simulink model "TosAeb"  (R2021a)
settings: Solver=FixedStepDiscrete  StartTime=0.0  StopTime=inf  FixedStep=0.01  RelTol=1e-3
undefined symbols (not defined in the file or in its folder): ACCModSts, AccSts1, AEB_ObjectMovingOrientation, AEB_ObjectMovingState, CN_BTCActive, CN_CurrentGearR, CN_CurrentGearR_DHT, CN_PTCActive, CN_VDCActive, CN_VSC_EspOff, CN_VSC_FCW_AEB_Error, CN_VSC_FCW_AEB_NoDispay, CN_VSC_FCW_AEB_PerfmDgdtn, CN_VSC_FCW_AEB_TempUnAvlbl, CN_VSC_VehStandstill, CNaccelAvoidMax, CNaccelAvoidMin, CNbrakePedalPress, CNcomfortStrLeftAccelMax, CNcomfortStrLeftAccelMin, CNcomfortStrRightAccelMax, CNcomfortStrRightAccelMin, CNDrvDoorOpen, CNDrvModeEco, CNDrvModeNorm, CNDrvModeOther, CNDrvModeSport, CNDrvSBRreminder, CNNull, CNobjInvalidID, CNPassDoorOpen, CNPassSBRreminder, CNPEBSAccelNegInf, CNPEBSAccelNull, CNPEBSTimeInf, CNPEBSTimeNull, CNSTOPMAX, CNSTOPNULL, CNsubjWidth, CNSwtOFF, CNSwtON, CNTTCMAX, CNTTCNULL, CNVehConfigAEBCycNone, CNVehConfigAEBNone, CNVehConfigAEBPedNone, CNVehConfigFCWNone, CNVehConfigJANone, CollisionSeverity, FALSE, FCWSnvty, JACycObjCalcInfoDefaultParams, JAPedObjCalcInfoDefaultParams, JAVehObjCalcInfoDefaultParams, latCycObjCalcInfoDefaultParams, latPedObjCalcInfoDefaultParams, longObjCalcInfoDefaultParams, ModSts, movingStatus, ObjectType ... (+1112)
libraries: simulink x3031, AEBSFunctionLib x50, Ta_Library x33, AEBSStateMachineLib x23, Mylibrary x12, simulink_extras x7, ObjSelect_lib x3, dspstat3 x2
(part 16 of 102)

== system TosAeb/FcwAebJa_Step_sys/ALG_AEBSystem/ALG_AEBController/AEB_LatObjInfoCalcApp/LatCycObjCalc/LatCycObjCalc ==
  latCycObjInfo : Inport  OutDataTypeStr=Bus: BusAEBLatObj  PortDimensions=1  Partition=on  PartitionDimension=1  PartitionWidth=1  PartitionOffset=0  BusOutputAsStruct=on
  subjInfo : Inport  Port=2  OutDataTypeStr=Bus: BusSubjInfo  PortDimensions=1
  Bus Selector : BusSelector  OutputSignals=subjVel
  Compare To Constant : simulink/Logic and Bit Operations/Compare To Constant  relop===  const=FALSE  OutDataTypeStr=boolean
  For Each : ForEach  DisableCoverage=on
  If : If  IfExpression=u1
  Merge : Merge
  TgtPreSelectCalc : Subsystem  TreatAsAtomicUnit=on
  latCycObjInfoCalc : Subsystem  TreatAsAtomicUnit=on
  latCycObjInfoDefault : Subsystem  TreatAsAtomicUnit=on
  latCycCalcInfo : Outport  OutDataTypeStr=Bus: BusLatCycObjCalcInfo  ConcatenationDimension=1
  ObjPreSelectCond : Outport  Port=2  ConcatenationDimension=1
  wires:
  latCycObjInfoDefault/1(latCycCalcInfo) -> Merge/1
  latCycObjInfo/1 -> TgtPreSelectCalc/1(latCycObjInfo), latCycObjInfoCalc/2(latCycObjInfo)  "latCycObjInfo"
  subjInfo/1 -> Bus Selector/1, latCycObjInfoCalc/1(subjInfo)
  If/2 -> latCycObjInfoCalc/Action
  If/1 -> latCycObjInfoDefault/Action
  latCycObjInfoCalc/1(latCycCalcInfo) -> Merge/2
  Merge/1 -> latCycCalcInfo/1
  Bus Selector/1 -> TgtPreSelectCalc/2(subjVel)  "<subjVel>"
  TgtPreSelectCalc/1(ObjPreSelectCond) -> ObjPreSelectCond/1, Compare To Constant/1
  Compare To Constant/1 -> If/1

== system TosAeb/FcwAebJa_Step_sys/ALG_AEBSystem/ALG_AEBController/AEB_LatObjInfoCalcApp/LatCycObjCalc/TargetSelectCalc ==
  latCycCalcInfo : Inport
  ObjPreSelectCond : Inport  Port=2
  Bus Selector : BusSelector  OutputSignals=latCycObjRawInfo.ObjectID
  P_LatCycCA_SelectObjCollisionRiskProbThres : Constant  Value=P_AEB_LatCycCA_SelectObjCollisionRiskProbThres
  P_LatCycCA_SelectTgtCollisionRiskProbThres : Constant  Value=P_AEB_LatCycCA_SelectTgtCollisionRiskProbThres
  Terminator : Terminator
  Terminator1 : Terminator
  Unit Delay : UnitDelay  SampleTime=-1  HasFrameUpgradeWarning=on
      settings: AttributesFormatString=InitialCondition:%<InitialCondition>
  tgtObjFlow1 : Stateflow chart  TreatAsAtomicUnit=on
  latCycObjCalcInfo : Outport
  wires:
  Unit Delay/1 -> tgtObjFlow1/5(TargetIDK1)
  tgtObjFlow1/2(tgtIsFind) -> Terminator/1
  tgtObjFlow1/1(tgtObj) -> Bus Selector/1, latCycObjCalcInfo/1  "latCycObjCalcInfo"
  Bus Selector/1 -> Unit Delay/1  "<ObjectID>"
  ObjPreSelectCond/1 -> tgtObjFlow1/2(ObjPreSelectCondFufilled)
  P_LatCycCA_SelectObjCollisionRiskProbThres/1 -> tgtObjFlow1/3(P_LatCycCA_SelectObjCollisionRiskProbThres)
  P_LatCycCA_SelectTgtCollisionRiskProbThres/1 -> tgtObjFlow1/4(P_LatCycCA_SelectTgtCollisionRiskProbThres)
  latCycCalcInfo/1 -> tgtObjFlow1/1(latCycCalcInfo)
  tgtObjFlow1/3(tgtIndex) -> Terminator1/1

== system TosAeb/FcwAebJa_Step_sys/ALG_AEBSystem/ALG_AEBController/AEB_LatObjInfoCalcApp/LatPedObjCalc/LatPedCollisonAssessInfo ==
  ObjectID : Inport
  pedColliisonRisk : Inport  Port=2  OutDataTypeStr=single
  dxFrontBumper : Inport  Port=3  OutDataTypeStr=single
  vx : Inport  Port=4
  subjVel : Inport  Port=5
  subLongAccel : Inport  Port=6  OutDataTypeStr=single
  FcwHMISetting : Inport  Port=7  OutDataTypeStr=Enum: FCWSnvty
  If : If  IfExpression=u1
  LatPedCollisonAssessInfo : Subsystem  TreatAsAtomicUnit=on
  LatPedCollisonAssessInfoDefault : Subsystem  TreatAsAtomicUnit=on
  Merge : Merge
  ObjIsInvalidJudge : Subsystem
  latPedCollisonAssessInfo : Outport
  wires:
  ObjIsInvalidJudge/1(ObjIsInvalid) -> If/1
  If/1 -> LatPedCollisonAssessInfoDefault/Action
  If/2 -> LatPedCollisonAssessInfo/Action
  ObjectID/1 -> LatPedCollisonAssessInfo/1(ObjectID), ObjIsInvalidJudge/1(ObjectID)
  pedColliisonRisk/1 -> LatPedCollisonAssessInfo/2(pedColliisonRisk)
  dxFrontBumper/1 -> LatPedCollisonAssessInfo/3(dxFrontBumper)
  subLongAccel/1 -> LatPedCollisonAssessInfo/6(subLongAccel)
  subjVel/1 -> LatPedCollisonAssessInfo/5(subjVel)
  FcwHMISetting/1 -> LatPedCollisonAssessInfo/7(FcwHMISetting)
  LatPedCollisonAssessInfo/1(latPedCollisonAssessInfo) -> Merge/2
  Merge/1 -> latPedCollisonAssessInfo/1
  LatPedCollisonAssessInfoDefault/1(latPedCollisonAssessInfo) -> Merge/1
  vx/1 -> LatPedCollisonAssessInfo/4(vx)

== system TosAeb/FcwAebJa_Step_sys/ALG_AEBSystem/ALG_AEBController/AEB_LatObjInfoCalcApp/LatPedObjCalc/LatPedObjCalc ==
  latPedObjInfo : Inport  OutDataTypeStr=Bus: BusAEBLatObj  PortDimensions=1  Partition=on  PartitionDimension=1  PartitionWidth=1  PartitionOffset=0  BusOutputAsStruct=on
  subjInfo : Inport  Port=2  OutDataTypeStr=Bus: BusSubjInfo  PortDimensions=1
  Bus Selector : BusSelector  OutputSignals=subjVel
  Compare To Constant : simulink/Logic and Bit Operations/Compare To Constant  relop===  const=FALSE  OutDataTypeStr=boolean
  For Each : ForEach  DisableCoverage=on
  If : If  IfExpression=u1
  Merge : Merge
  ObjPreSelectCondCalc : Subsystem
  latPedObjInfoCalc : Subsystem  TreatAsAtomicUnit=on
  latPedObjInfoDefault : Subsystem  TreatAsAtomicUnit=on
  latPedCalcInfo : Outport  OutDataTypeStr=Bus: BusLatPedObjCalcInfo  ConcatenationDimension=1
  ObjPreSelectCond : Outport  Port=2  ConcatenationDimension=1
  wires:
  latPedObjInfoCalc/1(latPedCalcInfo) -> Merge/2
  If/1 -> latPedObjInfoDefault/Action
  latPedObjInfo/1 -> latPedObjInfoCalc/2(latPedObjInfo), ObjPreSelectCondCalc/1(latPedObjInfo)  "latPedObjInfo"
  If/2 -> latPedObjInfoCalc/Action
  latPedObjInfoDefault/1(latPedCalcInfo) -> Merge/1
  Merge/1 -> latPedCalcInfo/1
  subjInfo/1 -> Bus Selector/1, latPedObjInfoCalc/1(subjInfo)  "subjInfo"
  Bus Selector/1 -> ObjPreSelectCondCalc/2(subjVel)  "<subjVel>"
  ObjPreSelectCondCalc/1(ObjPreSelectCond) -> ObjPreSelectCond/1, Compare To Constant/1
  Compare To Constant/1 -> If/1

== system TosAeb/FcwAebJa_Step_sys/ALG_AEBSystem/ALG_AEBController/AEB_LatObjInfoCalcApp/LatPedObjCalc/Subsystem ==
  latPedObjInfo : Inport  OutDataTypeStr=Bus: BusAEBLatObj  PortDimensions=1  BusOutputAsStruct=on
  subjInfo : Inport  Port=2  OutDataTypeStr=Bus: BusSubjInfo  PortDimensions=1
  Bus Selector : BusSelector  OutputSignals=subjVel
  Compare To Constant : simulink/Logic and Bit Operations/Compare To Constant  relop===  const=FALSE  OutDataTypeStr=boolean
  If : If  IfExpression=u1
  Merge : Merge
  ObjPreSelectCondCalc : Subsystem
  latPedObjInfoCalc : Subsystem  TreatAsAtomicUnit=on
  latPedObjInfoDefault : Subsystem  TreatAsAtomicUnit=on
  latPedCalcInfo : Outport  OutDataTypeStr=Bus: BusLatPedObjCalcInfo
  ObjPreSelectCond : Outport  Port=2
  wires:
  latPedObjInfoCalc/1(latPedCalcInfo) -> Merge/2
  Merge/1 -> latPedCalcInfo/1
  If/2 -> latPedObjInfoCalc/Action
  subjInfo/1 -> Bus Selector/1, latPedObjInfoCalc/1(subjInfo)  "subjInfo"
  latPedObjInfo/1 -> latPedObjInfoCalc/2(latPedObjInfo), ObjPreSelectCondCalc/1(latPedObjInfo)  "latPedObjInfo"
  ObjPreSelectCondCalc/1(ObjPreSelectCond) -> ObjPreSelectCond/1, Compare To Constant/1
  Bus Selector/1 -> ObjPreSelectCondCalc/2(subjVel)  "<subjVel>"
  latPedObjInfoDefault/1(latPedCalcInfo) -> Merge/1
  Compare To Constant/1 -> If/1
  If/1 -> latPedObjInfoDefault/Action

== system TosAeb/FcwAebJa_Step_sys/ALG_AEBSystem/ALG_AEBController/AEB_LatObjInfoCalcApp/LatPedObjCalc/TargetSelectCalc ==
  latPedCalcInfo : Inport  OutDataTypeStr=Bus: BusLatPedObjCalcInfo
  ObjPreSelectCond : Inport  Port=2
  Bus Selector1 : BusSelector  OutputSignals=latPedObjRawInfo.ObjectID
  P_LatPedCA_SelectObjCollisionRiskProbThres : Constant  Value=P_AEB_LatPedCA_SelectObjCollisionRiskProbThres
  P_LatPedCA_SelectTgtCollisionRiskProbThres : Constant  Value=P_AEB_LatPedCA_SelectTgtCollisionRiskProbThres
  Terminator1 : Terminator
  Terminator2 : Terminator
  Unit Delay2 : UnitDelay  SampleTime=-1  HasFrameUpgradeWarning=on
      settings: AttributesFormatString=InitialCondition:%<InitialCondition>
  tgtObjFlow1 : Stateflow chart  TreatAsAtomicUnit=on
  latPedObjCalcInfo : Outport
  wires:
  tgtObjFlow1/1(tgtObj) -> Bus Selector1/1, latPedObjCalcInfo/1
  ObjPreSelectCond/1 -> tgtObjFlow1/2(ObjPreSelectCondFufilled)
  tgtObjFlow1/3(tgtIsFind) -> Terminator1/1
  Bus Selector1/1 -> Unit Delay2/1  "<ObjectID>"
  Unit Delay2/1 -> tgtObjFlow1/5(TargetIDK1)
  latPedCalcInfo/1 -> tgtObjFlow1/1(latPedCalcInfo)
  P_LatPedCA_SelectObjCollisionRiskProbThres/1 -> tgtObjFlow1/3(P_LatPedCA_SelectObjCollisionRiskProbThres)
  P_LatPedCA_SelectTgtCollisionRiskProbThres/1 -> tgtObjFlow1/4(P_LatPedCA_SelectTgtCollisionRiskProbThres)
  tgtObjFlow1/2(tgtIndex) -> Terminator2/1

== system TosAeb/FcwAebJa_Step_sys/ALG_AEBSystem/ALG_AEBController/AEB_LongTgtInfoCalc/LongObjCalcInfoCalc/AEBS_LongCollisionAssesApp ==
  note: AEBS Longitude Collision Assess System
  ACCState : Inport
  FcwHMISetting : Inport  Port=2  OutDataTypeStr=Enum: FCWSnvty
  longObjInfo : Inport  Port=3  OutDataTypeStr=Bus: BusAEBLongObj
  longObjAbsInfo : Inport  Port=4  OutDataTypeStr=Bus: BusLongObjAbsInfo
  vehCanInfo : Inport  Port=5  OutDataTypeStr=Bus: BusVehCanInfo
  subjInfo : Inport  Port=6  OutDataTypeStr=Bus: BusSubjInfo
  Bus Creator : BusCreator  Inputs=6
  Bus Creator2 : BusCreator  Inputs=13  OutDataTypeStr=Bus: BusLongCollisionAssess
      settings: InheritFromInputs=off
  Bus Creator3 : BusCreator  Inputs=2
  Bus Selector : BusSelector  OutputSignals=subjInfo.subjLongAccel,subjInfo.subjVel,longObjAbsInfo.dxFrontBumper,longObjAbsInfo.axAbsObj,longObjAbsInfo.vxAbsObj,longObjInfo.ax,longObjInfo.vx
  Bus Selector1 : BusSelector  OutputSignals=ACCState,vehCanInfo.BrakePedalStatus_G,vehCanInfo.ESP_MasterCylindBrakePress,vehCanInfo.AccrPedlPosnDiagc,vehCanInfo.SteerWheelSpd,vehCanInfo.LeftTurnSWSts,vehCanInfo.RightTurnSWSts,FcwHMISetting
  Bus Selector12 : BusSelector  OutputSignals=longCollisionAvoidAssess.lowLvlBrkFlg4Warning,longCollisionAvoidAssess.highLvlBrkFlg4Warning,latAvoidAssess.latAvoidFlg4Warning
  Bus Selector13 : BusSelector  OutputSignals=longCollisionAvoidAssess.lowLvlBrkFlg4Brake,longCollisionAvoidAssess.highLvlBrkFlg4Brake,latAvoidAssess.latAvoidFlg4Brake
  Bus Selector14 : BusSelector  OutputSignals=longObjInfo.ObjectMovingState,longObjInfo.vx,longObjAbsInfo.dxFrontBumper,longObjAbsInfo.vxAbsObj,longObjAbsInfo.axAbsObj,subjInfo.subjLongAccel,subjInfo.subjVel,FcwHMISetting,longObjInfo.ObjectID
  Bus Selector15 : BusSelector  OutputSignals=TTC
  Bus Selector18 : BusSelector  OutputSignals=subjInfo.subjVel,longObjInfo.ObjectMovingState,longObjInfo.dy,longObjAbsInfo.dxFrontBumper,longObjInfo.vx,longObjInfo.vy,FcwHMISetting
  Bus Selector2 : BusSelector  OutputSignals=longCollisionAvoidAssess.accelAvoidLowLvlBrk,longCollisionAvoidAssess.lowLvlBrkFlg4Warning,longCollisionAvoidAssess.lowLvlBrkFlg4Brake,longCollisionAvoidAssess.accelAvoidHighLvlBrk4Warning,longCollisionAvoidAssess.highLvlBrkFlg4Warning,longCollisionAvoidAssess.accelAvoidHighLvlBrk4Brake,longCollisionAvoidAssess.highLvlBrkFlg4Brake,latAvoidAssess.accelLatAvoid.accelLatAvoidLeft,latAvoidAssess.accelLatAvoid.accelLatAvoidRight,latAvoidAssess.latAvoidFlg4Warning,latAvoidAssess.latAvoidFlg4Brake
  Bus Selector3 : BusSelector  OutputSignals=longObjInfo.vx,longObjAbsInfo.axAbsObj,longObjAbsInfo.vxAbsObj,longObjInfo.ax,longObjAbsInfo.dxFrontBumper,subjInfo.subjVel,subjInfo.subjLongAccel,FcwHMISetting
  Bus Selector5 : BusSelector  OutputSignals=tEgoStop,tObjStop,TTC
  Bus Selector8 : BusSelector  OutputSignals=TTC
  DriverReactionTimeCalc : Subsystem  TreatAsAtomicUnit=on
  LatCollisionAvoidCalc : Subsystem  TreatAsAtomicUnit=on
  LongCollisionAvoidAssess : Subsystem  TreatAsAtomicUnit=on
  LongCollisionSeverityCalc : Subsystem  TreatAsAtomicUnit=on
  TTCCalc : Subsystem  TreatAsAtomicUnit=on
  collisionSeverityHighCalc : Subsystem  TreatAsAtomicUnit=on
  collisionSeverityLowCalc : Subsystem  TreatAsAtomicUnit=on
  collisionSeverityMediumCalc : Subsystem  TreatAsAtomicUnit=on
  LongCollisionAssess : Outport  OutDataTypeStr=Bus: BusLongCollisionAssess
  wires:
  Bus Selector1/7 -> DriverReactionTimeCalc/7(RightTurnSWSts)  "<RightTurnSWSts>"
  Bus Selector18/5 -> LatCollisionAvoidCalc/6(vx)  "<vx>"
  Bus Selector14/6 -> collisionSeverityHighCalc/7(subjLongAccel)  "<subjLongAccel>"
  subjInfo/1 -> Bus Creator/6  "subjInfo"
  LongCollisionSeverityCalc/1(longCollisionSeverity) -> Bus Creator2/1  "longCollisionSeverity"
  Bus Selector3/3 -> LongCollisionAvoidAssess/7(vxAbsObj)  "<vxAbsObj>"
  longObjInfo/1 -> Bus Creator/3  "longObjInfo"
  collisionSeverityMediumCalc/1(collisionSeverityMediumFlg) -> LongCollisionSeverityCalc/2(collisionSeverityMediumFlg), collisionSeverityHighCalc/1(collisionSeverityMediumFlg)
  Bus Selector14/3 -> collisionSeverityHighCalc/4(dxFrontBumper)  "<dxFrontBumper>"
  Bus Selector1/4 -> DriverReactionTimeCalc/4(AccrPedlPosnDiagc)  "<AccrPedlPosnDiagc>"
  Bus Selector5/1 -> LongCollisionAvoidAssess/1(tEgoStop)  "<tEgoStop>"
  Bus Selector18/3 -> LatCollisionAvoidCalc/4(dy)  "<dy>"
  Bus Selector/6 -> TTCCalc/6(ax)  "<ax>"
  Bus Selector12/2 -> collisionSeverityLowCalc/2(highLvlBrkFlg4Warning)  "<highLvlBrkFlg4Warning>"
  Bus Selector/7 -> TTCCalc/7(vx)  "<vx>"
  LongCollisionAvoidAssess/1(longCollisionAvoidAssess) -> Bus Creator3/1  "longCollisionAvoidAssess"
  ACCState/1 -> Bus Creator/1  "ACCState"
  Bus Selector13/1 -> collisionSeverityMediumCalc/1(lowLvlBrkFlg4Brake)  "<lowLvlBrkFlg4Brake>"
  Bus Selector8/1 -> Bus Creator2/2  "<TTC>"
  Bus Selector3/4 -> LongCollisionAvoidAssess/8(ax)  "<ax>"
  Bus Selector3/8 -> LongCollisionAvoidAssess/12(FcwHMISetting)  "<FcwHMISetting>"
  LatCollisionAvoidCalc/1(latAvoidAssess) -> Bus Creator3/2  "latAvoidAssess"
  Bus Selector/4 -> TTCCalc/4(axAbsObj)  "<axAbsObj>"
  Bus Selector3/6 -> LongCollisionAvoidAssess/10(subjVel)  "<subjVel>"
  Bus Selector1/2 -> DriverReactionTimeCalc/2(BrakePedalStatus_G)  "<BrakePedalStatus_G>"
  Bus Selector/5 -> TTCCalc/5(vxAbsObj)  "<vxAbsObj>"
  Bus Selector14/1 -> collisionSeverityHighCalc/2(ObjectMovingState)  "<ObjectMovingState>"
  Bus Selector1/8 -> DriverReactionTimeCalc/8(FcwHMISetting)  "<FcwHMISetting>"
  Bus Creator/1 -> Bus Selector1/1, Bus Selector3/1, Bus Selector14/1, Bus Selector18/1, Bus Selector/1
  TTCCalc/1(TTCCalcOut) -> Bus Selector5/1, [TTCInfo]
  longObjAbsInfo/1 -> Bus Creator/4  "longObjAbsInfo"
  Bus Selector12/1 -> collisionSeverityLowCalc/1(lowLvlBrkFlg4Warning)  "<lowLvlBrkFlg4Warning>"
  Bus Creator3/1 -> [accelAvoidInfo], Bus Selector13/1, Bus Selector12/1
  Bus Selector18/7 -> LatCollisionAvoidCalc/8(FcwHMISetting)  "<FcwHMISetting>"
  Bus Selector3/7 -> LongCollisionAvoidAssess/11(subjLongAccel)  "<subjLongAccel>"
  Bus Selector18/2 -> LatCollisionAvoidCalc/3(ObjectMovingState)  "<ObjectMovingState>"
  Bus Selector1/6 -> DriverReactionTimeCalc/6(LeftTurnSWSts)  "<LeftTurnSWSts>"
  Bus Selector5/2 -> LongCollisionAvoidAssess/2(tObjStop)  "<tObjStop>"
  Bus Selector3/2 -> LongCollisionAvoidAssess/6(axAbsObj)  "<axAbsObj>"
  collisionSeverityHighCalc/1(collisionSeverityHighFlg) -> LongCollisionSeverityCalc/3(collisionSeverityHighFlg)
  Bus Selector12/3 -> collisionSeverityLowCalc/3(latAvoidFlg4Warning)  "<latAvoidFlg4Warning>"
  collisionSeverityLowCalc/1(collisionSeverityLowFlg) -> LongCollisionSeverityCalc/1(collisionSeverityLowFlg)
  Bus Selector14/5 -> collisionSeverityHighCalc/6(axAbsObj)  "<axAbsObj>"
  [TTCInfo] -> Bus Selector15/1
  Bus Selector14/2 -> collisionSeverityHighCalc/3(vx)  "<vx>"
  Bus Selector3/5 -> LongCollisionAvoidAssess/9(dxFrontBumper)  "<dxFrontBumper>"
  Bus Selector15/1 -> LatCollisionAvoidCalc/1(TTC)  "<TTC>"
  Bus Selector1/5 -> DriverReactionTimeCalc/5(SteerWheelSpd)  "<SteerWheelSpd>"
  [accelAvoidInfo] -> Bus Selector2/1
  Bus Selector13/2 -> collisionSeverityMediumCalc/2(highLvlBrkFlg4Brake)  "<highLvlBrkFlg4Brake>"
  Bus Selector18/1 -> LatCollisionAvoidCalc/2(subjVel)  "<subjVel>"
  Bus Selector1/1 -> DriverReactionTimeCalc/1(ACCState)  "<ACCState>"
  Bus Selector14/4 -> collisionSeverityHighCalc/5(vxAbsObj)  "<vxAbsObj>"
  Bus Creator2/1 -> LongCollisionAssess/1  "LongCollisionAssess"
  vehCanInfo/1 -> Bus Creator/5  "vehCanInfo"
  Bus Selector18/6 -> LatCollisionAvoidCalc/7(vy)  "<vy>"
  Bus Selector/1 -> TTCCalc/1(subjLongAccel)  "<subjLongAccel>"
  Bus Selector3/1 -> LongCollisionAvoidAssess/5(vx)  "<vx>"
  FcwHMISetting/1 -> Bus Creator/2  "FcwHMISetting"
  Bus Selector13/3 -> collisionSeverityMediumCalc/3(latAvoidFlg4Brake)  "<latAvoidFlg4Brake>"
  Bus Selector14/7 -> collisionSeverityHighCalc/8(subjVel)  "<subjVel>"
  Bus Selector14/9 -> LongCollisionSeverityCalc/4(ObjectID)  "<ObjectID>"
  Bus Selector/2 -> TTCCalc/2(subjVel)  "<subjVel>"
  Bus Selector1/3 -> DriverReactionTimeCalc/3(ESP_MasterCylindBrakePress)  "<ESP_MasterCylindBrakePress>"
  DriverReactionTimeCalc/1(diverReactionTime) -> LongCollisionAvoidAssess/4(tReaction)
  Bus Selector5/3 -> LongCollisionAvoidAssess/3(TTC)  "<TTC>"
  [TTCInfo] -> Bus Selector8/1
  Bus Selector/3 -> TTCCalc/3(dxFrontBumper)  "<dxFrontBumper>"
  Bus Selector18/4 -> LatCollisionAvoidCalc/5(dxFrontBumper)  "<dxFrontBumper>"
  Bus Selector14/8 -> collisionSeverityHighCalc/9(FcwHMISetting)  "<FcwHMISetting>"
  Bus Selector2/2 -> Bus Creator2/4  "<lowLvlBrkFlg4Warning>"
  Bus Selector2/1 -> Bus Creator2/3  "<accelAvoidLowLvlBrk>"
  Bus Selector2/3 -> Bus Creator2/5  "<lowLvlBrkFlg4Brake>"
  Bus Selector2/4 -> Bus Creator2/6  "<accelAvoidHighLvlBrk4Warning>"
  Bus Selector2/5 -> Bus Creator2/7  "<highLvlBrkFlg4Warning>"
  Bus Selector2/6 -> Bus Creator2/8  "<accelAvoidHighLvlBrk4Brake>"
  Bus Selector2/7 -> Bus Creator2/9  "<highLvlBrkFlg4Brake>"
  Bus Selector2/8 -> Bus Creator2/10  "<accelLatAvoidLeft>"
  Bus Selector2/9 -> Bus Creator2/11  "<accelLatAvoidRight>"
  Bus Selector2/10 -> Bus Creator2/12  "<latAvoidFlg4Warning>"
  Bus Selector2/11 -> Bus Creator2/13  "<latAvoidFlg4Brake>"

== system TosAeb/FcwAebJa_Step_sys/ALG_AEBSystem/ALG_AEBController/AEB_LongTgtInfoCalc/LongObjCalcInfoCalc/ObjAbsInfoTrans ==
  dx : Inport
  vx : Inport  Port=2
  ax : Inport  Port=3
  ObjectID : Inport  Port=4
  ObjectTypeCameraConfirmed : Inport  Port=5
  ObjectType : Inport  Port=6
  FR_ObjectID : Inport  Port=7
  FR_Dx : Inport  Port=8
  FR_Dy : Inport  Port=9
  FR_Vx : Inport  Port=10
  FC_ObjectID : Inport  Port=11
  FC_Dx : Inport  Port=12
  FC_Dy : Inport  Port=13
  FC_Vx : Inport  Port=14
  subjVel : Inport  Port=15
  subjLongAccel : Inport  Port=16
  Constant : Constant  Value=P_Global_DxvFrontBumper_m
  Constant1 : Constant  Value=0  OutDataTypeStr=single
  MinMax : MinMax  Function=max  Inputs=2
  ObjFusQualityCal : Stateflow chart  TreatAsAtomicUnit=on
  Subtract : Sum  Inputs=+-
  Subtract1 : Sum
  Subtract2 : Sum
  dxFrontBumper : Outport
  vxAbsObj : Outport  Port=2
  axAbsObj : Outport  Port=3
  ObjFusQuality : Outport  Port=4
  wires:
  dx/1 -> Subtract/1
  MinMax/1 -> dxFrontBumper/1  "dxFrontBumper"
  Subtract1/1 -> vxAbsObj/1  "vxAbsObj"
  ax/1 -> Subtract2/1
  subjLongAccel/1 -> Subtract2/2
  Subtract2/1 -> axAbsObj/1  "axAbsObj"
  Constant/1 -> Subtract/2
  Subtract/1 -> MinMax/1
  Constant1/1 -> MinMax/2
  ObjectID/1 -> ObjFusQualityCal/1(ObjectID)
  ObjectTypeCameraConfirmed/1 -> ObjFusQualityCal/2(ObjectTypeCameraConfirmed)
  FC_ObjectID/1 -> ObjFusQualityCal/4(FC_ObjectID)
  FR_ObjectID/1 -> ObjFusQualityCal/5(FR_ObjectID)
  FR_Dy/1 -> ObjFusQualityCal/7(FR_Dy)
  FR_Dx/1 -> ObjFusQualityCal/6(FR_Dx)
  FC_Dx/1 -> ObjFusQualityCal/9(FC_Dx)
  FC_Dy/1 -> ObjFusQualityCal/10(FC_Dy)
  vx/1 -> Subtract1/2, ObjFusQualityCal/12(Fus_Vx)
  subjVel/1 -> Subtract1/1, ObjFusQualityCal/13(subjVel)
  ObjFusQualityCal/1(ObjFusQuality) -> ObjFusQuality/1
  FC_Vx/1 -> ObjFusQualityCal/11(FC_Vx)
  FR_Vx/1 -> ObjFusQualityCal/8(FR_Vx)
  ObjectType/1 -> ObjFusQualityCal/3(Fus_ObjectType)

== system TosAeb/FcwAebJa_Step_sys/ALG_AEBSystem/ALG_AEBController/AEB_LongTgtInfoCalc/LongObjCalcInfoCalc/OverlapCalculation ==
  ObjectWidth : Inport
  ObjectTypeCameraConfirmed : Inport  Port=2
  ObjectMovingState : Inport  Port=3
  dyEgo2Center : Inport  Port=4
  dyObject2Center : Inport  Port=5
  OverlapRatioCalcFlow : Stateflow chart  TreatAsAtomicUnit=on
  TargetWidthCalc : Subsystem
  dyCriticalEgoObjCalc : Subsystem
  dyOverlapActualCalc1 : Subsystem  Commented=on
  overlapRatio : Outport
  wires:
  ObjectWidth/1 -> TargetWidthCalc/1(ObjectWidth)
  ObjectTypeCameraConfirmed/1 -> TargetWidthCalc/2(ObjectTypeCameraConfirmed)
  ObjectMovingState/1 -> TargetWidthCalc/3(ObjectMovingState)
  TargetWidthCalc/1(objWidth) -> dyCriticalEgoObjCalc/1(objWidth)  "objWidth"
  dyCriticalEgoObjCalc/1(dyCriticalEgoObj) -> OverlapRatioCalcFlow/1(dyCriticalEgoObj)  "dyCriticalEgoObj"
  OverlapRatioCalcFlow/1(overlapRatio) -> overlapRatio/1
  dyEgo2Center/1 -> OverlapRatioCalcFlow/2(dyEgo2Center)
  dyObject2Center/1 -> OverlapRatioCalcFlow/3(dyObject2Center)

== system TosAeb/FcwAebJa_Step_sys/ALG_AEBSystem/ALG_AEBController/AEB_LongTgtInfoCalc/LongObjCalcInfoCalc/ProbEscapeCalcu ==
  ObjectWidth : Inport
  ObjectTypeCameraConfirmed : Inport  Port=2
  ObjectMovingState : Inport  Port=3
  dy : Inport  Port=4
  vx : Inport  Port=5
  vy : Inport  Port=6
  dxFrontBumper : Inport  Port=7
  subjLatAccel : Inport  Port=8
  subjYawRate : Inport  Port=9
  subjVel : Inport  Port=10
  subjCurvTraj : Inport  Port=11
  Merge : Merge
  MovingObjdyEscapeCalcu : Subsystem  TreatAsAtomicUnit=on
  ProbEscapeCalc : Subsystem  TreatAsAtomicUnit=on
  StationaryObjEscapeCalcu : Subsystem  TreatAsAtomicUnit=on
  Subsystem : Subsystem  TreatAsAtomicUnit=on
  TargetIsStandingConfirm : Subsystem  TreatAsAtomicUnit=on
  TargetWidthCalc : Subsystem  TreatAsAtomicUnit=on
  objIsMovingTargetJudge : If  IfExpression=u1
  probEscape : Outport
  wires:
  ObjectMovingState/1 -> TargetWidthCalc/3(ObjectMovingState), TargetIsStandingConfirm/1(ObjectMovingState)  "ObjectMovingState"
  objIsMovingTargetJudge/1 -> StationaryObjEscapeCalcu/Action
  MovingObjdyEscapeCalcu/1(dyEscape) -> Merge/2
  StationaryObjEscapeCalcu/1(dyEscape) -> Merge/1
  objIsMovingTargetJudge/2 -> [MovingTrg]
  [MovingTrg] -> MovingObjdyEscapeCalcu/Action
  Merge/1 -> ProbEscapeCalc/2(dyEscape)  "dyEscape"
  ProbEscapeCalc/1(probEscape) -> probEscape/1  "probEscape"
  subjLatAccel/1 -> StationaryObjEscapeCalcu/2(subjLatAccel)
  subjVel/1 -> Subsystem/2(subjVel), MovingObjdyEscapeCalcu/1(subjVel)
  vx/1 -> MovingObjdyEscapeCalcu/3(vx)
  vy/1 -> MovingObjdyEscapeCalcu/4(vy)
  dxFrontBumper/1 -> Subsystem/1(dxFrontBumper), [dxFrontBumper]
  subjYawRate/1 -> MovingObjdyEscapeCalcu/5(subjYawRate)
  subjCurvTraj/1 -> MovingObjdyEscapeCalcu/6(subjCurvTraj)
  dy/1 -> StationaryObjEscapeCalcu/1(dy), MovingObjdyEscapeCalcu/2(dy)  "dy"
  TargetWidthCalc/1(ObjWidth) -> ProbEscapeCalc/1(ObjWidth)  "ObjWidth"
  ObjectWidth/1 -> TargetWidthCalc/1(ObjectWidth)  "ObjectWidth"
  ObjectTypeCameraConfirmed/1 -> TargetWidthCalc/2(ObjectTypeCameraConfirmed)  "FusionType"
  TargetIsStandingConfirm/1(TargetIsStandingFlg) -> objIsMovingTargetJudge/1
  Subsystem/1(Timegap) -> StationaryObjEscapeCalcu/3(TTC), [TTC]
  [TTC] -> MovingObjdyEscapeCalcu/8(TTC)
  [dxFrontBumper] -> MovingObjdyEscapeCalcu/7(dxFrontBumper)

== system TosAeb/FcwAebJa_Step_sys/ALG_AEBSystem/ALG_AEBController/AEB_StateMachineCalcApp/JA_AEBStateMachine/JA_PTW_AEBfullStateMachine ==
  inputSignal : Inport
  AEBFuncFaultMap : Subsystem
  AEBStateMachine : AEBSStateMachineLib/AEBStateMachine  SystemSampleTime=-1  VariantActivationTime=update diagram  Latency=0  AutoFrameSizeCalculation=off
  ActivationCondCalc : Subsystem
  ActiveStateAborCondCalc : Subsystem
  Bus Selector : BusSelector  OutputSignals=AVHStsActv,vehStateCalcInfo.VSC_FCW_AEB_FctSts.FCW_AEB_JAFctSts,vehStateCalcInfo.VSC_AEBFuncSwtSts.AEB_JAResp
  Constant : Constant  Value=P_AEB_FuncStatus_JaPedAEBfullWorkTime
  Constant1 : Constant  Value=P_AEB_FuncStatus_JaPedAEBfullStandstillWorkTime
  Constant{4..14} (11 alike) : Constant  Value=TRUE
  Constant2 : Constant  Value=Ts
  Constant3 : Constant  Value=FALSE
  StandstillStateAborCondCalc : Subsystem
  Terminator1 : Terminator
  JA_PTW_AEBRawModSts : Outport
  JA_PTW_AEBModSts : Outport  Port=2
  wires:
  Constant1/1 -> AEBStateMachine/10
  Constant2/1 -> AEBStateMachine/8
  Constant/1 -> AEBStateMachine/9
  ActivationCondCalc/1(ActivationCondFulfilled) -> AEBStateMachine/5
  ActiveStateAborCondCalc/1(ActiveStateAborCondFulfilled) -> AEBStateMachine/6
  Bus Selector/3 -> AEBStateMachine/3  "<AEB_JAResp>"
  Constant3/1 -> AEBStateMachine/4
  inputSignal/1 -> Bus Selector/1
  Bus Selector/2 -> AEBFuncFaultMap/1(FCW_AEB_VehFctSts)  "<FCW_AEB_JAFctSts>"
  StandstillStateAborCondCalc/1(ActiveStateAborCondFulfilled) -> AEBStateMachine/7
  AEBStateMachine/3 -> Terminator1/1
  AEBStateMachine/2 -> JA_PTW_AEBModSts/1
  AEBStateMachine/1 -> JA_PTW_AEBRawModSts/1
  Constant5/1 -> ActivationCondCalc/1(JA_PTW_AEBfullSuppCond)
  Constant6/1 -> ActivationCondCalc/2(JA_PTW_AEBfullSuppArb)
  Constant7/1 -> ActivationCondCalc/3(PTW_AEBfullFuncImpSuppCond)
  Constant8/1 -> ActiveStateAborCondCalc/1(JA_PTW_AEBfullAborCond)
  Constant9/1 -> ActiveStateAborCondCalc/2(JA_PTW_AEBfullAborArb)
  Constant10/1 -> ActiveStateAborCondCalc/3(JA_PTW_AEBfullFuncImpAborCond)
  Constant11/1 -> ActiveStateAborCondCalc/4(JABrkManeuAborCond)
  Constant13/1 -> StandstillStateAborCondCalc/1(JA_PTW_AEBfullAborCond)
  Constant14/1 -> StandstillStateAborCondCalc/2(JA_PTW_AEBfullAborArb)
  Constant4/1 -> StandstillStateAborCondCalc/3(JA_PTW_AEBfullFuncImpAborCond)
  Constant12/1 -> StandstillStateAborCondCalc/4(JABrkManeuAborCond)
  Bus Selector/1 -> AEBStateMachine/1  "<AVHStsActv>"
  AEBFuncFaultMap/1(Fault) -> AEBStateMachine/2

== system TosAeb/FcwAebJa_Step_sys/ALG_AEBSystem/ALG_AEBController/AEB_StateMachineCalcApp/JA_AEBStateMachine/JA_PTW_PrefillStateMachine ==
  inputSignal : Inport
  AEBFuncFaultMap : Subsystem
  AEBStateMachine : AEBSStateMachineLib/AEBStateMachine  SystemSampleTime=-1  VariantActivationTime=update diagram  Latency=0  AutoFrameSizeCalculation=off
  ActivationCondCalc : Subsystem
  ActiveStateAborCondCalc : Subsystem
  Bus Selector : BusSelector  OutputSignals=vehStateCalcInfo.VSC_FCW_AEB_FctSts.FCW_AEB_JAFctSts,vehStateCalcInfo.VSC_AEBFuncSwtSts.AEB_JAResp
  Constant : Constant  Value=P_AEB_FuncStatus_JaPedPrefillWorkTime
  Constant1 : Constant  Value=P_AEB_FuncStatus_JaPedPrefillStandstillWorkTime
  Constant{5..11} (7 alike) : Constant  Value=TRUE
  Constant12 : Constant  Value=FALSE
  Constant2 : Constant  Value=Ts
  Constant3 : Constant  Value=FALSE
  Constant4 : Constant  Value=FALSE
  Terminator : Terminator
  JA_PTW_PrefillRawModSts : Outport
  JA_PTW_PrefillModSts : Outport  Port=2
  wires:
  Constant/1 -> AEBStateMachine/9
  Constant2/1 -> AEBStateMachine/8
  Constant1/1 -> AEBStateMachine/10
  ActivationCondCalc/1(ActivationCondFulfilled) -> AEBStateMachine/5
  Constant3/1 -> AEBStateMachine/7
  inputSignal/1 -> Bus Selector/1
  Bus Selector/1 -> AEBFuncFaultMap/1(FCW_AEB_VehFctSts)  "<FCW_AEB_JAFctSts>"
  Constant4/1 -> AEBStateMachine/4
  Bus Selector/2 -> AEBStateMachine/3  "<AEB_JAResp>"
  ActiveStateAborCondCalc/1(ActiveStateAborCondFulfilled) -> AEBStateMachine/6
  AEBStateMachine/3 -> Terminator/1
  AEBStateMachine/2 -> JA_PTW_PrefillModSts/1
  AEBStateMachine/1 -> JA_PTW_PrefillRawModSts/1
  Constant5/1 -> ActivationCondCalc/1(JA_PTW_PrefillSuppCond)
  Constant6/1 -> ActivationCondCalc/2(JA_PTW_PrefillSuppArb)
  Constant7/1 -> ActivationCondCalc/3(PTW_PrefillFuncImpSuppCond)
  Constant8/1 -> ActiveStateAborCondCalc/1(JA_PTW_PrefillAborCond)
  Constant9/1 -> ActiveStateAborCondCalc/2(JA_PTW_PrefillAborArb)
  Constant10/1 -> ActiveStateAborCondCalc/3(PTW_PrefillFuncImpAborCond)
  Constant11/1 -> ActiveStateAborCondCalc/4(ABPManeuAborCond)
  Constant12/1 -> AEBStateMachine/1
  AEBFuncFaultMap/1(Fault) -> AEBStateMachine/2

== system TosAeb/FcwAebJa_Step_sys/ALG_AEBSystem/ALG_AEBController/AEB_StateMachineCalcApp/JA_AEBStateMachine/JA_cycAEBfullStateMachine ==
  inputSignal : Inport
  AEBFuncFaultMap : Subsystem
  AEBStateMachine : AEBSStateMachineLib/AEBStateMachine  SystemSampleTime=-1  VariantActivationTime=update diagram  Latency=0  AutoFrameSizeCalculation=off
  ActivationCondCalc : Subsystem
  ActiveStateAborCondCalc : Subsystem
  Bus Selector : BusSelector  OutputSignals=AVHStsActv,vehStateCalcInfo.VSC_FCW_AEB_FctSts.FCW_AEB_JAFctSts,vehStateCalcInfo.VSC_AEBFuncSwtSts.AEB_PedResp,vehStateCalcInfo.VSCVehStandStill
  Bus Selector1 : BusSelector  OutputSignals=JACycFuncSuppAborCond.JACycAEBfullSuppCond,JA_FctStsArbSuppAborCond.brkSuppAborCondArb.JA_cycAEBSuppArb,FuncImpSuppAborCond.brkFuncImpSuppAborCond.brkFuncImpAborCond.JA_cycAEBfullFuncImpAborCond
  Bus Selector2 : BusSelector  OutputSignals=JACycFuncSuppAborCond.JACycAEBfullAborCondNoStandstill,JA_FctStsArbSuppAborCond.brkSuppAborCondArb.JA_cycAEBAborArb,FuncImpSuppAborCond.brkFuncImpSuppAborCond.brkFuncImpAborCond.JA_cycAEBfullFuncImpAborCond,FuncManeuAborCond.JABrkManeuAborCond
  Bus Selector3 : BusSelector  OutputSignals=JACycFuncSuppAborCond.JACycAEBfullAborCondStandstill,JA_FctStsArbSuppAborCond.brkSuppAborCondArb.JA_cycAEBAborArb,FuncImpSuppAborCond.brkFuncImpSuppAborCond.brkFuncImpAborCond.JA_cycAEBfullFuncImpAborCond,FuncManeuAborCond.JABrkManeuAborCond
  Constant : Constant  Value=P_AEB_FuncStatus_JaCycAEBfullWorkTime
  Constant1 : Constant  Value=P_AEB_FuncStatus_JaCycAEBfullStandstillWorkTime
  Constant2 : Constant  Value=Ts
  StandstillStateAborCondCalc : Subsystem
  Terminator1 : Terminator
  JA_cycAEBRawModSts : Outport
  JA_cycAEBModSts : Outport  Port=2
  wires:
  AEBFuncFaultMap/1(Fault) -> AEBStateMachine/2
  Bus Selector/1 -> AEBStateMachine/1  "<AVHStsActv>"
  AEBStateMachine/1 -> JA_cycAEBRawModSts/1
  AEBStateMachine/2 -> JA_cycAEBModSts/1
  AEBStateMachine/3 -> Terminator1/1
  Bus Selector3/3 -> StandstillStateAborCondCalc/3(JA_cycAEBfullFuncImpAborCond)  "<JA_cycAEBfullFuncImpAborCond>"
  Bus Selector3/4 -> StandstillStateAborCondCalc/4(JABrkManeuAborCond)  "<JABrkManeuAborCond>"
  Bus Selector3/2 -> StandstillStateAborCondCalc/2(JA_cycAEBfullAborArb)  "<JA_cycAEBAborArb>"
  Bus Selector3/1 -> StandstillStateAborCondCalc/1(JA_cycAEBfullAborCond)  "<JACycAEBfullAborCondStandstill>"
  StandstillStateAborCondCalc/1(ActiveStateAborCondFulfilled) -> AEBStateMachine/7
  Bus Selector2/1 -> ActiveStateAborCondCalc/1(JA_cycAEBfullAborCond)  "<JACycAEBfullAborCondNoStandstill>"
  Bus Selector/2 -> AEBFuncFaultMap/1(FCW_AEB_VehFctSts)  "<FCW_AEB_JAFctSts>"
  inputSignal/1 -> Bus Selector/1, Bus Selector2/1, Bus Selector3/1, Bus Selector1/1
  Bus Selector1/3 -> ActivationCondCalc/3(JA_cycFuncImpSuppCond)  "<JA_cycAEBfullFuncImpAborCond>"
  Bus Selector2/2 -> ActiveStateAborCondCalc/2(JA_cycAEBfullAborArb)  "<JA_cycAEBAborArb>"
  Bus Selector1/1 -> ActivationCondCalc/1(JA_cycAEBfullSuppCond)  "<JACycAEBfullSuppCond>"
  Bus Selector1/2 -> ActivationCondCalc/2(JA_cycAEBfullSuppArb)  "<JA_cycAEBSuppArb>"
  Bus Selector/4 -> AEBStateMachine/4  "<VSCVehStandStill>"
  Bus Selector/3 -> AEBStateMachine/3  "<AEB_PedResp>"
  ActiveStateAborCondCalc/1(ActiveStateAborCondFulfilled) -> AEBStateMachine/6
  ActivationCondCalc/1(ActivationCondFulfilled) -> AEBStateMachine/5
  Bus Selector2/4 -> ActiveStateAborCondCalc/4(JABrkManeuAborCond)  "<JABrkManeuAborCond>"
  Bus Selector2/3 -> ActiveStateAborCondCalc/3(JA_cycAEBfullFuncImpAborCond)  "<JA_cycAEBfullFuncImpAborCond>"
  Constant/1 -> AEBStateMachine/9
  Constant2/1 -> AEBStateMachine/8
  Constant1/1 -> AEBStateMachine/10

== system TosAeb/FcwAebJa_Step_sys/ALG_AEBSystem/ALG_AEBController/AEB_StateMachineCalcApp/JA_AEBStateMachine/JA_cycPrefillStateMachine ==
  inputSignal : Inport
  AEBFuncFaultMap : Subsystem
  AEBStateMachine : AEBSStateMachineLib/AEBStateMachine  SystemSampleTime=-1  VariantActivationTime=update diagram  Latency=0  AutoFrameSizeCalculation=off
  ActivationCondCalc : Subsystem
  ActiveStateAborCondCalc : Subsystem
  Bus Selector : BusSelector  OutputSignals=vehStateCalcInfo.VSC_FCW_AEB_FctSts.FCW_AEB_JAFctSts,vehStateCalcInfo.VSC_AEBFuncSwtSts.AEB_PedResp
  Bus Selector1 : BusSelector  OutputSignals=JACycFuncSuppAborCond.JACycPrefillSuppCond,JA_FctStsArbSuppAborCond.brkReadySuppAborCondArb.JA_cycPrefillSuppArb,FuncImpSuppAborCond.brkReadyFuncImpSuppAborCond.brkReadyFuncImpSuppCond.JA_cycPrefillFuncImpSuppCond
  Bus Selector2 : BusSelector  OutputSignals=JAPedFuncSuppAborCond.JAPedPrefillAborCond,JA_FctStsArbSuppAborCond.brkReadySuppAborCondArb.JA_cycPrefillAborArb,FuncImpSuppAborCond.brkReadyFuncImpSuppAborCond.brkReadyFuncImpAborCond.JA_cycPrefillFuncImpAborCond,FuncManeuAborCond.ABPFuncManeuAborCond
  Constant : Constant  Value=P_AEB_FuncStatus_JaCycPrefillWorkTime
  Constant1 : Constant  Value=P_AEB_FuncStatus_JaCycPrefillStandstillWorkTime
  Constant2 : Constant  Value=Ts
  Constant3 : Constant  Value=FALSE
  Constant4 : Constant  Value=FALSE
  Constant5 : Constant  Value=FALSE
  Terminator : Terminator
  JA_cycPrefillRawModSts : Outport
  JA_cycPrefillModSts : Outport  Port=2
  wires:
  AEBFuncFaultMap/1(Fault) -> AEBStateMachine/2
  Constant5/1 -> AEBStateMachine/1
  AEBStateMachine/1 -> JA_cycPrefillRawModSts/1
  AEBStateMachine/2 -> JA_cycPrefillModSts/1
  AEBStateMachine/3 -> Terminator/1
  Bus Selector2/4 -> ActiveStateAborCondCalc/4(ABPManeuAborCond)  "<ABPFuncManeuAborCond>"
  Bus Selector2/3 -> ActiveStateAborCondCalc/3(JA_cycFuncImpAborCond)  "<JA_cycPrefillFuncImpAborCond>"
  Bus Selector2/2 -> ActiveStateAborCondCalc/2(JA_cycPrefillAborArb)  "<JA_cycPrefillAborArb>"
  Bus Selector2/1 -> ActiveStateAborCondCalc/1(JA_cycPrefillAborCond)  "<JAPedPrefillAborCond>"
  ActiveStateAborCondCalc/1(ActiveStateAborCondFulfilled) -> AEBStateMachine/6
  Bus Selector1/3 -> ActivationCondCalc/3(JA_cycPrefillFuncImpSuppCond)  "<JA_cycPrefillFuncImpSuppCond>"
  Bus Selector1/2 -> ActivationCondCalc/2(JA_cycPrefillSuppArb)  "<JA_cycPrefillSuppArb>"
  Bus Selector/2 -> AEBStateMachine/3  "<AEB_PedResp>"
  Constant4/1 -> AEBStateMachine/4
  Bus Selector1/1 -> ActivationCondCalc/1(JA_cycPrefillSuppCond)  "<JACycPrefillSuppCond>"
  Bus Selector/1 -> AEBFuncFaultMap/1(FCW_AEB_VehFctSts)  "<FCW_AEB_JAFctSts>"
  inputSignal/1 -> Bus Selector/1, Bus Selector1/1, Bus Selector2/1
  Constant3/1 -> AEBStateMachine/7
  ActivationCondCalc/1(ActivationCondFulfilled) -> AEBStateMachine/5
  Constant1/1 -> AEBStateMachine/10
  Constant2/1 -> AEBStateMachine/8
  Constant/1 -> AEBStateMachine/9

== system TosAeb/FcwAebJa_Step_sys/ALG_AEBSystem/ALG_AEBController/AEB_StateMachineCalcApp/JA_AEBStateMachine/JA_pedAEBfullStateMachine ==
  inputSignal : Inport
  AEBFuncFaultMap : Subsystem
  AEBStateMachine : AEBSStateMachineLib/AEBStateMachine  SystemSampleTime=-1  VariantActivationTime=update diagram  Latency=0  AutoFrameSizeCalculation=off
  ActivationCondCalc : Subsystem
  ActiveStateAborCondCalc : Subsystem
  Bus Selector : BusSelector  OutputSignals=AVHStsActv,vehStateCalcInfo.VSC_FCW_AEB_FctSts.FCW_AEB_JAFctSts,vehStateCalcInfo.VSC_AEBFuncSwtSts.AEB_PedResp,vehStateCalcInfo.VSCVehStandStill
  Bus Selector1 : BusSelector  OutputSignals=JAPedFuncSuppAborCond.JAPedAEBfullSuppCond,JA_FctStsArbSuppAborCond.brkSuppAborCondArb.JA_pedAEBSuppArb,FuncImpSuppAborCond.brkFuncImpSuppAborCond.brkFuncImpSuppCond.JA_pedAEBfullFuncImpSuppCond
  Bus Selector2 : BusSelector  OutputSignals=JAPedFuncSuppAborCond.JAPedAEBfullAborCondNoStandstill,JA_FctStsArbSuppAborCond.brkSuppAborCondArb.JA_pedAEBAborArb,FuncImpSuppAborCond.brkFuncImpSuppAborCond.brkFuncImpAborCond.JA_pedAEBfullFuncImpAborCond,FuncManeuAborCond.JABrkManeuAborCond
  Bus Selector3 : BusSelector  OutputSignals=JAPedFuncSuppAborCond.JAPedAEBfullAborCondStandstill,JA_FctStsArbSuppAborCond.brkSuppAborCondArb.JA_pedAEBAborArb,FuncImpSuppAborCond.brkFuncImpSuppAborCond.brkFuncImpAborCond.JA_pedAEBfullFuncImpAborCond,FuncManeuAborCond.JABrkManeuAborCond
  Constant : Constant  Value=P_AEB_FuncStatus_JaPedAEBfullWorkTime
  Constant1 : Constant  Value=P_AEB_FuncStatus_JaPedAEBfullStandstillWorkTime
  Constant2 : Constant  Value=Ts
  StandstillStateAborCondCalc : Subsystem
  Terminator1 : Terminator
  JA_pedAEBRawModSts : Outport
  JA_pedAEBModSts : Outport  Port=2
  wires:
  Constant1/1 -> AEBStateMachine/10
  Constant2/1 -> AEBStateMachine/8
  Constant/1 -> AEBStateMachine/9
  Bus Selector2/3 -> ActiveStateAborCondCalc/3(JA_pedAEBfullFuncImpAborCond)  "<JA_pedAEBfullFuncImpAborCond>"
  Bus Selector2/4 -> ActiveStateAborCondCalc/4(JABrkManeuAborCond)  "<JABrkManeuAborCond>"
  ActivationCondCalc/1(ActivationCondFulfilled) -> AEBStateMachine/5
  ActiveStateAborCondCalc/1(ActiveStateAborCondFulfilled) -> AEBStateMachine/6
  Bus Selector/3 -> AEBStateMachine/3  "<AEB_PedResp>"
  Bus Selector/4 -> AEBStateMachine/4  "<VSCVehStandStill>"
  Bus Selector1/2 -> ActivationCondCalc/2(JA_pedAEBfullSuppArb)  "<JA_pedAEBSuppArb>"
  Bus Selector1/1 -> ActivationCondCalc/1(JA_pedAEBfullSuppCond)  "<JAPedAEBfullSuppCond>"
  Bus Selector2/2 -> ActiveStateAborCondCalc/2(JA_pedAEBfullAborArb)  "<JA_pedAEBAborArb>"
  inputSignal/1 -> Bus Selector1/1, Bus Selector3/1, Bus Selector2/1, Bus Selector/1
  Bus Selector/2 -> AEBFuncFaultMap/1(FCW_AEB_VehFctSts)  "<FCW_AEB_JAFctSts>"
  Bus Selector2/1 -> ActiveStateAborCondCalc/1(JA_pedAEBfullAborCond)  "<JAPedAEBfullAborCondNoStandstill>"
  StandstillStateAborCondCalc/1(ActiveStateAborCondFulfilled) -> AEBStateMachine/7
  Bus Selector3/1 -> StandstillStateAborCondCalc/1(JA_pedAEBfullAborCond)  "<JAPedAEBfullAborCondStandstill>"
  Bus Selector3/2 -> StandstillStateAborCondCalc/2(JA_pedAEBfullAborArb)  "<JA_pedAEBAborArb>"
  Bus Selector3/4 -> StandstillStateAborCondCalc/4(JABrkManeuAborCond)  "<JABrkManeuAborCond>"
  Bus Selector3/3 -> StandstillStateAborCondCalc/3(JA_pedAEBfullFuncImpAborCond)  "<JA_pedAEBfullFuncImpAborCond>"
  AEBStateMachine/3 -> Terminator1/1
  AEBStateMachine/2 -> JA_pedAEBModSts/1
  AEBStateMachine/1 -> JA_pedAEBRawModSts/1
  Bus Selector/1 -> AEBStateMachine/1  "<AVHStsActv>"
  AEBFuncFaultMap/1(Fault) -> AEBStateMachine/2
  Bus Selector1/3 -> ActivationCondCalc/3(JA_pedAEBfullFuncImpSuppCond)  "<JA_pedAEBfullFuncImpSuppCond>"

== system TosAeb/FcwAebJa_Step_sys/ALG_AEBSystem/ALG_AEBController/AEB_StateMachineCalcApp/JA_AEBStateMachine/JA_pedPrefillStateMachine ==
  inputSignal : Inport
  AEBFuncFaultMap : Subsystem
  AEBStateMachine : AEBSStateMachineLib/AEBStateMachine  SystemSampleTime=-1  VariantActivationTime=update diagram  Latency=0  AutoFrameSizeCalculation=off
  ActivationCondCalc : Subsystem
  ActiveStateAborCondCalc : Subsystem
  Bus Selector : BusSelector  OutputSignals=vehStateCalcInfo.VSC_FCW_AEB_FctSts.FCW_AEB_JAFctSts,vehStateCalcInfo.VSC_AEBFuncSwtSts.AEB_PedResp
  Bus Selector1 : BusSelector  OutputSignals=JAPedFuncSuppAborCond.JAPedPrefillSuppCond,JA_FctStsArbSuppAborCond.brkReadySuppAborCondArb.JA_pedPrefillSuppArb,FuncImpSuppAborCond.brkReadyFuncImpSuppAborCond.brkReadyFuncImpSuppCond.JA_pedPrefillFuncImpSuppCond
  Bus Selector2 : BusSelector  OutputSignals=JAPedFuncSuppAborCond.JAPedPrefillAborCond,JA_FctStsArbSuppAborCond.brkSuppAborCondArb.JA_pedAEBAborArb,FuncImpSuppAborCond.brkReadyFuncImpSuppAborCond.brkReadyFuncImpAborCond.JA_pedPrefillFuncImpAborCond,FuncManeuAborCond.ABPFuncManeuAborCond
  Constant : Constant  Value=P_AEB_FuncStatus_JaPedPrefillWorkTime
  Constant1 : Constant  Value=P_AEB_FuncStatus_JaPedPrefillStandstillWorkTime
  Constant2 : Constant  Value=Ts
  Constant3 : Constant  Value=FALSE
  Constant4 : Constant  Value=FALSE
  Constant5 : Constant  Value=FALSE
  Terminator : Terminator
  JA_pedPrefillRawModSts : Outport
  JA_pedPrefillModSts : Outport  Port=2
  wires:
  Constant/1 -> AEBStateMachine/9
  Constant2/1 -> AEBStateMachine/8
  Constant1/1 -> AEBStateMachine/10
  ActivationCondCalc/1(ActivationCondFulfilled) -> AEBStateMachine/5
  Constant3/1 -> AEBStateMachine/7
  inputSignal/1 -> Bus Selector2/1, Bus Selector1/1, Bus Selector/1
  Bus Selector/1 -> AEBFuncFaultMap/1(FCW_AEB_VehFctSts)  "<FCW_AEB_JAFctSts>"
  Bus Selector1/1 -> ActivationCondCalc/1(JA_pedPrefillSuppCond)  "<JAPedPrefillSuppCond>"
  Constant4/1 -> AEBStateMachine/4
  Bus Selector/2 -> AEBStateMachine/3  "<AEB_PedResp>"
  Bus Selector1/2 -> ActivationCondCalc/2(JA_pedPrefillSuppArb)  "<JA_pedPrefillSuppArb>"
  Bus Selector1/3 -> ActivationCondCalc/3(JA_pedPrefillFuncImpSuppCond)  "<JA_pedPrefillFuncImpSuppCond>"
  ActiveStateAborCondCalc/1(ActiveStateAborCondFulfilled) -> AEBStateMachine/6
  Bus Selector2/1 -> ActiveStateAborCondCalc/1(JA_pedPrefillAborCond)  "<JAPedPrefillAborCond>"
  Bus Selector2/2 -> ActiveStateAborCondCalc/2(JA_pedPrefillAborArb)  "<JA_pedAEBAborArb>"
  Bus Selector2/3 -> ActiveStateAborCondCalc/3(JA_pedPrefillFuncImpAborCond)  "<JA_pedPrefillFuncImpAborCond>"
  Bus Selector2/4 -> ActiveStateAborCondCalc/4(ABPManeuAborCond)  "<ABPFuncManeuAborCond>"
  AEBStateMachine/3 -> Terminator/1
  AEBStateMachine/2 -> JA_pedPrefillModSts/1
  AEBStateMachine/1 -> JA_pedPrefillRawModSts/1
  Constant5/1 -> AEBStateMachine/1
  AEBFuncFaultMap/1(Fault) -> AEBStateMachine/2

== system TosAeb/FcwAebJa_Step_sys/ALG_AEBSystem/ALG_AEBController/AEB_StateMachineCalcApp/JA_AEBStateMachine/JA_vehAEBStateMachine ==
  inputSignal : Inport
  AEBFuncFaultMap : Subsystem
  AEBStateMachine : AEBSStateMachineLib/AEBStateMachine  SystemSampleTime=-1  VariantActivationTime=update diagram  Latency=0  AutoFrameSizeCalculation=off
  ActivationCondCalc : Subsystem
  ActiveStateAborCondCalc : Subsystem
  Bus Selector : BusSelector  OutputSignals=AVHStsActv,vehStateCalcInfo.VSC_FCW_AEB_FctSts.FCW_AEB_JAFctSts,vehStateCalcInfo.VSC_AEBFuncSwtSts.AEB_JAResp,vehStateCalcInfo.VSCVehStandStill
  Bus Selector1 : BusSelector  OutputSignals=JAVehFuncSuppAborCond.JAVehAEBfullSuppCond,JA_FctStsArbSuppAborCond.brkSuppAborCondArb.JA_vehAEBSuppArb,FuncImpSuppAborCond.brkFuncImpSuppAborCond.brkFuncImpSuppCond.JA_vehAEBfullFuncImpSuppCond
  Bus Selector2 : BusSelector  OutputSignals=JAVehFuncSuppAborCond.JAVehAEBfullAborCondNoStandstill,JA_FctStsArbSuppAborCond.brkSuppAborCondArb.JA_vehAEBAborArb,FuncImpSuppAborCond.brkFuncImpSuppAborCond.brkFuncImpAborCond.JA_vehAEBfullFuncImpAborCond,FuncManeuAborCond.JABrkManeuAborCond
  Bus Selector3 : BusSelector  OutputSignals=JAVehFuncSuppAborCond.JAVehAEBfullAborCondStandstill,JA_FctStsArbSuppAborCond.brkSuppAborCondArb.JA_vehAEBAborArb,FuncImpSuppAborCond.brkFuncImpSuppAborCond.brkFuncImpAborCond.JA_vehAEBfullFuncImpAborCond,FuncManeuAborCond.JABrkManeuAborCond
  Constant : Constant  Value=P_AEB_FuncStatus_JaVehAEBfullWorkTime
  Constant1 : Constant  Value=P_AEB_FuncStatus_JaVehAEBfullStandstillWorkTime
  Constant2 : Constant  Value=Ts
  StandstillStateAborCondCalc : Subsystem
  Terminator : Terminator
  JA_vehAEBRawModSts : Outport
  JA_vehAEBModSts : Outport  Port=2
  wires:
  Constant1/1 -> AEBStateMachine/10
  Constant2/1 -> AEBStateMachine/8
  Constant/1 -> AEBStateMachine/9
  Bus Selector/2 -> AEBFuncFaultMap/1(FCW_AEB_VehFctSts)  "<FCW_AEB_JAFctSts>"
  ActiveStateAborCondCalc/1(ActiveStateAborCondFulfilled) -> AEBStateMachine/6
  inputSignal/1 -> Bus Selector1/1, Bus Selector3/1, Bus Selector2/1, Bus Selector/1
  ActivationCondCalc/1(ActivationCondFulfilled) -> AEBStateMachine/5
  Bus Selector1/2 -> ActivationCondCalc/2(JA_vehAEBfullSuppArb)  "<JA_vehAEBSuppArb>"
  Bus Selector1/3 -> ActivationCondCalc/3(JA_vehAEBfullFuncImpSuppCond)  "<JA_vehAEBfullFuncImpSuppCond>"
  Bus Selector/3 -> AEBStateMachine/3  "<AEB_JAResp>"
  Bus Selector1/1 -> ActivationCondCalc/1(JA_vehAEBfullSuppCond)  "<JAVehAEBfullSuppCond>"
  Bus Selector2/1 -> ActiveStateAborCondCalc/1(JA_vehAEBfullAborCondNoStandstill)  "<JAVehAEBfullAborCondNoStandstill>"
  Bus Selector2/2 -> ActiveStateAborCondCalc/2(JA_vehAEBfullAborArb)  "<JA_vehAEBAborArb>"
  Bus Selector2/3 -> ActiveStateAborCondCalc/3(JA_vehAEBfullFuncImpAborCond)  "<JA_vehAEBfullFuncImpAborCond>"
  Bus Selector2/4 -> ActiveStateAborCondCalc/4(JABrkManeuAborCond)  "<JABrkManeuAborCond>"
  Bus Selector/4 -> AEBStateMachine/4  "<VSCVehStandStill>"
  Bus Selector3/1 -> StandstillStateAborCondCalc/1(JA_vehAEBfullAborCondStandstill)  "<JAVehAEBfullAborCondStandstill>"
  Bus Selector3/2 -> StandstillStateAborCondCalc/2(JA_vehAEBfullAborArb)  "<JA_vehAEBAborArb>"
  Bus Selector3/3 -> StandstillStateAborCondCalc/3(JA_vehAEBfullFuncImpAborCond)  "<JA_vehAEBfullFuncImpAborCond>"
  Bus Selector3/4 -> StandstillStateAborCondCalc/4(JABrkManeuAborCond)  "<JABrkManeuAborCond>"
  StandstillStateAborCondCalc/1(ActiveStateAborCondFulfilled) -> AEBStateMachine/7
  AEBStateMachine/3 -> Terminator/1
  AEBStateMachine/2 -> JA_vehAEBModSts/1  "JA_vehAEBModSts"
  AEBStateMachine/1 -> JA_vehAEBRawModSts/1
  Bus Selector/1 -> AEBStateMachine/1  "<AVHStsActv>"
  AEBFuncFaultMap/1(Fault) -> AEBStateMachine/2

== system TosAeb/FcwAebJa_Step_sys/ALG_AEBSystem/ALG_AEBController/AEB_StateMachineCalcApp/JA_AEBStateMachine/JA_vehPrefillStateMachine ==
  inputSignal : Inport
  AEBFuncFaultMap : Subsystem
  AEBStateMachine : AEBSStateMachineLib/AEBStateMachine  SystemSampleTime=-1  VariantActivationTime=update diagram  Latency=0  AutoFrameSizeCalculation=off
  ActivationCondCalc : Subsystem
  ActiveStateAborCondCalc : Subsystem
  Bus Selector : BusSelector  OutputSignals=vehStateCalcInfo.VSC_FCW_AEB_FctSts.FCW_AEB_JAFctSts,vehStateCalcInfo.VSC_AEBFuncSwtSts.AEB_JAResp
  Bus Selector1 : BusSelector  OutputSignals=JAVehFuncSuppAborCond.JAVehPrefillSuppCond,JA_FctStsArbSuppAborCond.brkReadySuppAborCondArb.JA_vehPrefillSuppArb,FuncImpSuppAborCond.brkReadyFuncImpSuppAborCond.brkReadyFuncImpSuppCond.JA_vehPrefillFuncImpSuppCond
  Bus Selector2 : BusSelector  OutputSignals=JAVehFuncSuppAborCond.JAVehPrefillAborCond,JA_FctStsArbSuppAborCond.brkReadySuppAborCondArb.JA_vehPrefillSuppArb,FuncImpSuppAborCond.brkReadyFuncImpSuppAborCond.brkReadyFuncImpAborCond.JA_vehPrefillFuncImpAborCond,FuncManeuAborCond.ABPFuncManeuAborCond
  Constant : Constant  Value=P_AEB_FuncStatus_JaVehPrefillWorkTime
  Constant1 : Constant  Value=P_AEB_FuncStatus_JaVehPrefillStandstillWorkTime
  Constant2 : Constant  Value=Ts
  Constant3 : Constant  Value=FALSE
  Constant4 : Constant  Value=FALSE
  Constant5 : Constant  Value=FALSE
  Terminator : Terminator
  JA_vehPrefillRawModSts : Outport
  JA_vehPrefillModSts : Outport  Port=2
  wires:
  Constant/1 -> AEBStateMachine/9
  Constant2/1 -> AEBStateMachine/8
  Constant1/1 -> AEBStateMachine/10
  Constant4/1 -> AEBStateMachine/4
  Bus Selector/2 -> AEBStateMachine/3  "<AEB_JAResp>"
  inputSignal/1 -> Bus Selector2/1, Bus Selector1/1, Bus Selector/1
  Bus Selector1/1 -> ActivationCondCalc/1(JA_vehPrefillSuppCond)  "<JAVehPrefillSuppCond>"
  Bus Selector/1 -> AEBFuncFaultMap/1(FCW_AEB_VehFctSts)  "<FCW_AEB_JAFctSts>"
  ActivationCondCalc/1(ActivationCondFulfilled) -> AEBStateMachine/5
  ActiveStateAborCondCalc/1(ActiveStateAborCondFulfilled) -> AEBStateMachine/6
  Bus Selector1/2 -> ActivationCondCalc/2(JA_vehPrefillSuppArb)  "<JA_vehPrefillSuppArb>"
  Constant3/1 -> AEBStateMachine/7
  Bus Selector1/3 -> ActivationCondCalc/3(JA_vehPrefillFuncImpSuppCond)  "<JA_vehPrefillFuncImpSuppCond>"
  Bus Selector2/1 -> ActiveStateAborCondCalc/1(JA_vehPrefillAborCond)  "<JAVehPrefillAborCond>"
  Bus Selector2/2 -> ActiveStateAborCondCalc/2(JA_vehPrefillAborArb)  "<JA_vehPrefillSuppArb>"
  Bus Selector2/3 -> ActiveStateAborCondCalc/3(JA_vehPrefillFuncImpAborCond)  "<JA_vehPrefillFuncImpAborCond>"
  Bus Selector2/4 -> ActiveStateAborCondCalc/4(ABPManeuAborCond)  "<ABPFuncManeuAborCond>"
  AEBStateMachine/3 -> Terminator/1
  AEBStateMachine/2 -> JA_vehPrefillModSts/1
  AEBStateMachine/1 -> JA_vehPrefillRawModSts/1
  Constant5/1 -> AEBStateMachine/1
  AEBFuncFaultMap/1(Fault) -> AEBStateMachine/2
